simulink model "Simulador_Lazaro_RL_F3"  (R2025b)
settings: Solver=ode45  StartTime=0.0  StopTime=6  FixedStep=0.02  RelTol=1e-3  EnableMultiTasking=on
model workspace (MAT data in the model file):
  ActorLearningRate = 0.0001
  CHECKPOINT_EVERY = 100
  CriticLearningRate = 0.0007
  Expl_Decay = 1.155238628e-05
  Expl_LowerLim = -0.5
  Expl_Mean = 0
  Expl_StdDev = 0.2
  Expl_StdDevMin = 0.05
  Expl_Steps = 120000
  Expl_UpperLim = 0.5
  IdRewardList = {'17'}
  LearningRate = 0.0001
  MAX_STEPS_EPISODE = 600
  SampleTime = 0.01
  ScenarioID = '32'
  TOTAL_EPISODES = 200
  Tgt_Decay = 0
  Tgt_LowerLim = -0.5
  Tgt_Mean = 0
  Tgt_StdDev = 0.2
  Tgt_StdDevMin = 0.2
  Tgt_Steps = 0
  Tgt_UpperLim = 0.5
  Ts = 0.01
  agent : rl.agent.rlTD3Agent (value not decoded)
undefined symbols (not defined in the file): DiscIntNeverNeededParam
libraries: simulink x2, rllib x1, slpidlib x1
settings Model: Creator=Administrador  LastModifiedBy=richards
settings SolverCC: ZeroCrossControl=DisableAll  AlgebraicLoopSolver=TrustRegion
settings DataIOCC: LimitDataPoints=on  SaveFormat=Array  SignalLoggingSaveFormat=ModelDataLogs  DSMLogging=off  ReturnWorkspaceOutputs=off
settings OptimizationCC: ExpressionDepthLimit=2147483647.0  LifeSpan=inf
settings DebuggingCC: UnderspecifiedInitializationDetection=Classic  MergeDetectMultiDrivingBlocksExec=none  SolverPrmCheckMsg=warning  FcnCallInpInsideContextMsg=warning  UnconnectedInputMsg=warning  UnconnectedOutputMsg=warning  UnconnectedLineMsg=warning  StateNameClashWarn=warning  SFUnexpectedBacktrackingDiag=warning  SFNoUnconditionalDefaultTransitionDiag=warning  SFExecutionAtInitializationDiag=none  ArithmeticOperatorsInVariantConditions=warning
settings HardwareCC: ProdBitPerPointer=32.0  ProdBitPerSizeT=32.0  ProdBitPerPtrDiffT=32.0  ProdLargestAtomicFloat=None  ProdIntDivRoundTo=Undefined  ProdEndianess=Unspecified  ProdWordSize=32.0  ProdHWDeviceType=32-bit Generic
settings ModelReferenceCC: EnableRefExpFcnMdlSchedulingChecks=on  PropagateSignalLabelsOutOfModel=off
settings SFSimCC: SimUseLocalCustomCode=off  SimParseCustomCode=off  CustomCodeUndefinedFunction=UseInterfaceOnly
settings RTWCC: RTWUseLocalCustomCode=off  GPUComputeCapability=3.5
settings CodeAppCC: StateflowObjectComments=on
settings GRTTargetCC: TargetLangStandard=C89/C90 (ANSI)  CombineOutputUpdateFcns=off  GRTInterface=on
settings ConfigComp: CovMetricSettings=dw  CovSaveCumulativeToWorkspaceVar=on  CovSaveSingleToWorkspaceVar=on  CovExternalEMLEnable=off

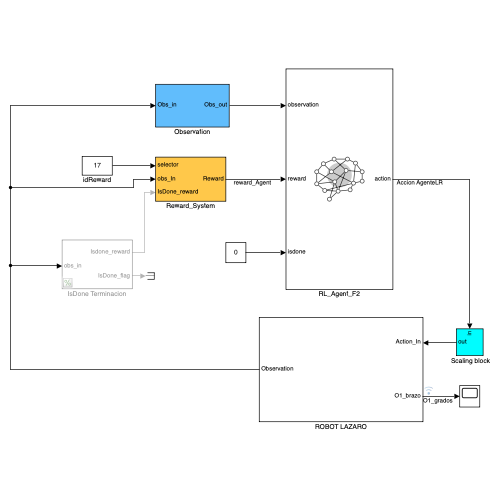
[thumbnail: Simulink's stored preview of the top-level diagram]
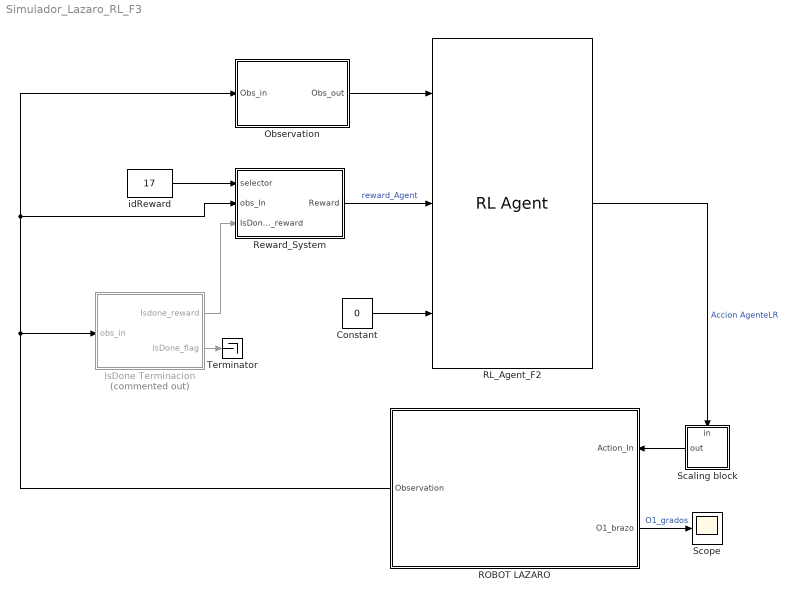
== system Simulador_Lazaro_RL_F3 ==
  " Scaling block" : Subsystem
      settings: PortSchema="{\"entries\":[{\"content\":{\"sides\":[{\"content\":{\"connectorIds\":[\"Out1\"]},\"type\":\"ConnectorPlacement.EquallySpacedRectSide\",\"uuid\":\"a790eab9-2b43-47a4-b062-d830449fd16c\"},{\"content\":{\"side\":\"RIGHT\"},\"type\":\"ConnectorPlacement.EquallySpacedRectSide\",\"uuid\":\"29b5b92f-6241-42c9-b69f-7e34ffae78d0\"},{\"content\":{\"connectorIds\":[\"In1\"],\"side\":\"TOP\"},\"type\":\"ConnectorPlacement.EquallySpacedRectSide\",\"uuid\":\"0d9138d4-6546-416f-9897-aa48eddc1957\"},{\"content\":{\"side\":\"BOTTOM\"},\"type\":\"ConnectorPlacement.EquallySpacedRectSide\",\"uuid\":\"c130b2a4-31b4-4ea6-a38d-9072e793f24d\"}]},\"type\":\"ConnectorPlacement.EquallySpacedConnector\",\"uuid\":\"38341b86-6b15-4731-82e8-a6b530589507\"}],\"packageUris\":[\"http://schema.mathworks.com/mf0/Connector/R2020b\"],\"version\":\"1.0\"}"
  Constant : Constant  Value=0
  IsDone Terminacion : Subsystem  Commented=on
  Observation : Subsystem
      settings: MinAlgLoopOccurrences=on
  RL_Agent_F2 : rllib/RL Agent  Agent=agent  ExternalActionAsInput=off  ProvideLastAction=off  ProvideCumRwd=off  TreatAsDirectFeedthrough=on  UseStrictObservationDataTypes=off
  ROBOT LAZARO : Subsystem
      settings: PortSchema="{\"entries\":[{\"content\":{\"sides\":[{\"content\":{\"connectorIds\":[\"Out2\"]},\"type\":\"ConnectorPlacement.EquallySpacedRectSide\",\"uuid\":\"37102e5d-7d53-4887-99df-ea23d4bb6b5f\"},{\"content\":{\"connectorIds\":[\"In1\",\"Out1\"],\"side\":\"RIGHT\"},\"type\":\"ConnectorPlacement.EquallySpacedRectSide\",\"uuid\":\"afcf0875-52fe-459c-9e77-306d435328c8\"},{\"content\":{\"side\":\"TOP\"},\"type\":\"ConnectorPlacement.EquallySpacedRectSide\",\"uuid\":\"2ef0c81c-cb20-4dc7-992a-873989b99f1b\"}]},\"type\":\"ConnectorPlacement.EquallySpacedConnector\",\"uuid\":\"5a61918d-759d-4f32-b21d-c30e6bf5e1c6\"}],\"packageUris\":[\"http://schema.mathworks.com/mf0/Connector/R2020b\"],\"version\":\"1.0\"}"
  Reward_System : Subsystem
  Scope : Scope
      settings: ActiveDisplayYMinimum=-0.33259239487088316  ActiveDisplayYMaximum=1.9531277507122846  DataLoggingVariableName=ScopeData10
  Terminator : Terminator
  idReward : Constant  Value=17  OutDataTypeStr=uint8
  wires:
  ROBOT LAZARO/2(Observation) -> Observation/1(Obs_in), Reward_System/2(obs_In), IsDone Terminacion/1(obs_in)
  ROBOT LAZARO/1(O1_brazo) -> Scope/1  "O1_grados"
  RL_Agent_F2/1 -> " Scaling block"/1(in)  "Accion AgenteLR"
  " Scaling block"/1(out) -> ROBOT LAZARO/1(Action_In)
  idReward/1 -> Reward_System/1(selector)
  Constant/1 -> RL_Agent_F2/3
  Reward_System/1(Reward) -> RL_Agent_F2/2  "reward_Agent"
  Observation/1(Obs_out) -> RL_Agent_F2/1
  IsDone Terminacion/1(Isdone_reward) -> Reward_System/3(IsDone_reward)
  IsDone Terminacion/2(IsDone_flag) -> Terminator/1

== system Simulador_Lazaro_RL_F3/ Scaling block ==
  note: Saturation:
      | upper: 20
      | lower: -20
  in : Inport
  Constant1 : Constant  Value=0
  K_RL : Gain  Gain=10
  Saturation : Saturate  UpperLimit=20  LowerLimit=-20
  Sum1 : Sum  Inputs=|++
  out : Outport
  wires:
  Sum1/1 -> out/1  "Tt"
  Saturation/1 -> Sum1/1  "K_RL_sat"
  in/1 -> K_RL/1
  Constant1/1 -> Sum1/2
  K_RL/1 -> Saturation/1  "K_RL"

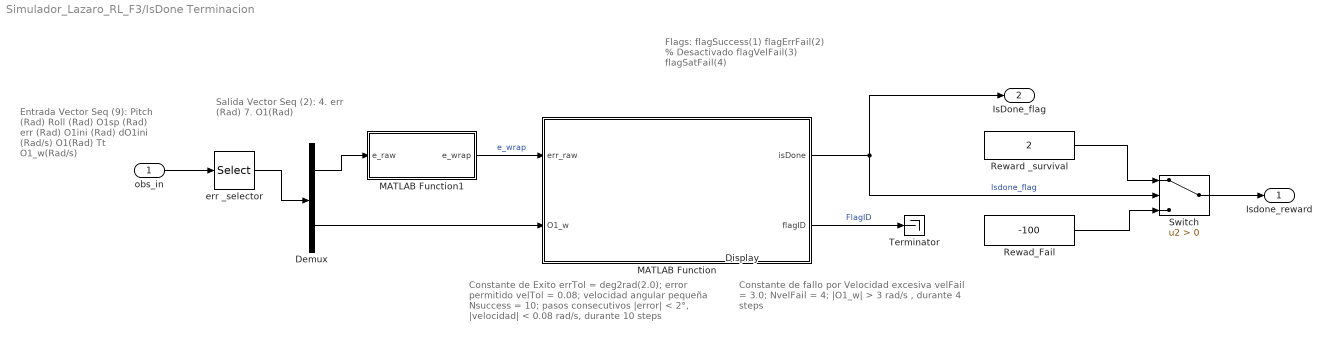
== system Simulador_Lazaro_RL_F3/IsDone Terminacion ==
  note: Flags:
      | flagSuccess(1)
      | flagErrFail(2) % Desactivado
      | flagVelFail(3)
      | flagSatFail(4)
  note: Constante de Exito
      |
      | errTol = deg2rad(2.0); error permitido
      | velTol = 0.08; velocidad angular pequeña
      | Nsuccess = 10; pasos consecutivos
      |
      | |error| < 2°, |velocidad| < 0.08 rad/s, durante 10 steps
  note: Constante de fallo por Velocidad excesiva
      |
      | velFail = 3.0;
      | NvelFail = 4;
      |
      | |O1_w| > 3 rad/s , durante 4 steps
  note: Entrada
      | Vector Seq (9):
      | Pitch (Rad)
      | Roll (Rad)
      | O1sp (Rad)
      | err (Rad)
      | O1ini (Rad)
      | dO1ini (Rad/s)
      | O1(Rad)
      | Tt
      | O1_w(Rad/s)
  note: Salida
      | Vector Seq (2):
      | 4. err (Rad)
      | 7. O1(Rad)
  obs_in : Inport
  Demux : Demux  Outputs=2
  Display : DisplayBlock  LabelPosition=Hide  Transparency=1  GridColor=[0.502, 0.502, 0.502]
  MATLAB Function : MATLAB Function  TreatAsAtomicUnit=on
  MATLAB Function1 : MATLAB Function  TreatAsAtomicUnit=on
  Rewad_Fail : Constant  Value=-100
  Reward _survival : Constant  Value=2
  Switch : Switch  Criteria=u2 > Threshold  SaturateOnIntegerOverflow=off  InputSameDT=off
  Terminator : Terminator
  err _selector : Selector  InputPortWidth=9  IndexOptions=Index vector (dialog)  Indices=[4 7]  OutputSizes=1
  Isdone_reward : Outport
  IsDone_flag : Outport  Port=2
  wires:
  obs_in/1 -> err _selector/1
  err _selector/1 -> Demux/1
  MATLAB Function1/1(e_wrap) -> MATLAB Function/1(err_raw)  "e_wrap"
  Rewad_Fail/1 -> Switch/3
  Switch/1 -> Isdone_reward/1
  MATLAB Function/1(isDone) -> IsDone_flag/1, Switch/2  "Isdone_flag"
  Reward _survival/1 -> Switch/1
  Demux/1 -> MATLAB Function1/1(e_raw)
  Demux/2 -> MATLAB Function/2(O1_w)
  MATLAB Function/2(flagID) -> Terminator/1  "FlagID"

== system Simulador_Lazaro_RL_F3/Observation ==
  note: Entrada:
  note: Vector de Observation:
      | Vector Seq (14):
      |
      | Pitch (sin)
      | Pitch (cos)
      | Roll (sin)
      | Roll (cos)
      | O1sp (sin)
      | O1sp (cos)
      | err (sin) / wrapeado
      | err (cos) / wrapeado
      | O1ini (sin)
      | O1ini (cos)
      | dO1ini (rad/s)
      | O1(sin)
      | O1(cos)
      | O1_w(Rad/s)
  note: Salida:
  note: Vector Seq (9):
      | Pitch (Rad)
      | Roll (Rad)
      | O1sp (Rad)
      | err (Rad)
      | O1ini (Rad)
      | dO1ini (Rad/s)
      | O1(Rad)
      | Tt
      | O1_vel(Rad/s)
  note: Vector Seq (5):
      | 1. Pitch (Rad)
      | 2. Roll (Rad)
      | 3. O1sp (Rad)
      | 5. O1ini (Rad)
      | 7. O1(Rad)
  note: Vector de Observation:
      | Vector Seq (2):
      | 6. dO1ini (Rad/s)
      | 9. O1_w(Rad/s)
  note: Salida:
      | Vector Seq (1):
      | 4. err (Rad)
  Obs_in : Inport
  Demux : Demux  Outputs=2
  MATLAB Function2 : MATLAB Function  TreatAsAtomicUnit=on
  Memory : Memory
  Selector : Selector  InputPortWidth=9  IndexOptions=Index vector (dialog)  Indices=[1 2 3 5 7]  OutputSizes=1
  Selector1 : Selector  InputPortWidth=9  IndexOptions=Index vector (dialog)  Indices=[6 9]  OutputSizes=1
  Selector2 : Selector  InputPortWidth=9  IndexOptions=Index vector (dialog)  Indices=[4]  OutputSizes=1
  Obs_out : Outport
  wires:
  Selector/1 -> MATLAB Function2/1(u_select)
  Obs_in/1 -> Selector/1, Selector1/1, Selector2/1
  Selector2/1 -> MATLAB Function2/2(err_raw)
  Selector1/1 -> Demux/1
  Demux/2 -> MATLAB Function2/4(O1_w)
  Demux/1 -> MATLAB Function2/3(dO1ini)
  MATLAB Function2/1(obs) -> Memory/1
  Memory/1 -> Obs_out/1

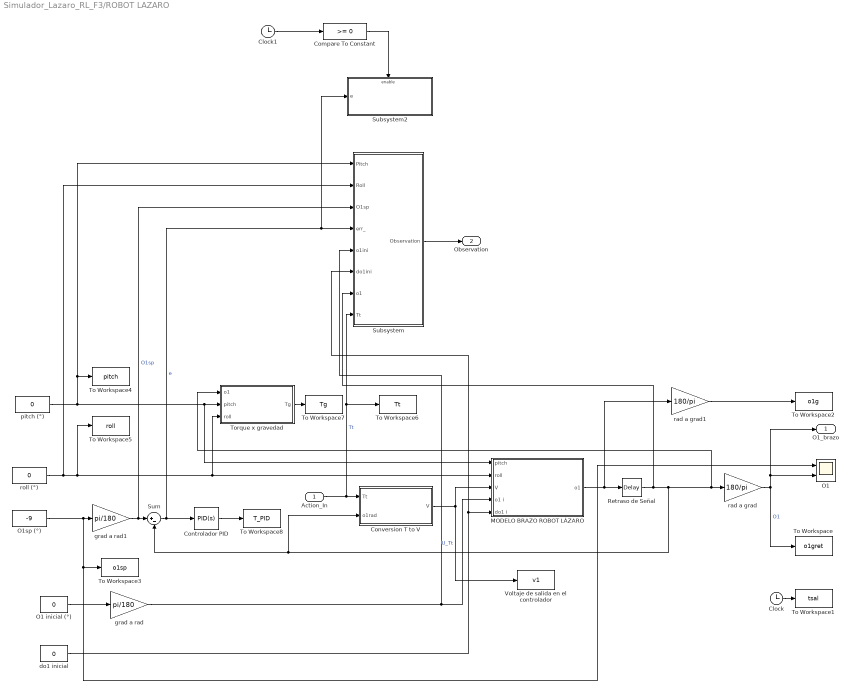
== system Simulador_Lazaro_RL_F3/ROBOT LAZARO ==
  Action_In : Inport
  Clock : Clock
  Clock1 : Clock
  Compare To Constant : simulink/Logic and Bit Operations/Compare To Constant  relop=>=  const=0  OutDataTypeStr=boolean
  Controlador PID : slpidlib/PID Controller  Controller=PID  Form=Parallel  TimeDomain=Continuous-time  UseExternalTs=off  SampleTime=1  IntegratorMethod=Forward Euler  FilterMethod=Forward Euler  ControllerParametersSource=internal  P=5  I=0.2  UseKiTs=off  D=2  UseExternalDerivativeSource=off  N=100  UseFilter=on  TunerSelectOption=Transfer Function Based (PID Tuner App)  InitialConditionSource=internal  InitialConditionForIntegrator=0  InitialConditionForFilter=0  DifferentiatorICPrevScaledInput=0  ExternalReset=none  TrackingMode=off  Kt=1  LimitOutput=off  SatLimitsSource=internal  UpperSaturationLimit=inf  LowerSaturationLimit=-inf  LinearizeAsGain=off  AntiWindupMode=none  Kb=1  LimitIntegrator=off  UpperIntegratorSaturationLimit=inf  LowerIntegratorSaturationLimit=-inf  POutMin=[]  POutMax=[]  IOutMin=[]  IOutMax=[]  DOutMin=[]  DOutMax=[]  NOutMin=[]  NOutMax=[]  SumOutMin=[]  SumOutMax=[]  SaturationOutMin=[]  SaturationOutMax=[]  PParamMin=[]  PParamMax=[]  IParamMin=[]  IParamMax=[]  DParamMin=[]  DParamMax=[]  NParamMin=[]  NParamMax=[]  KbParamMin=[]  KbParamMax=[]  KtParamMin=[]  KtParamMax=[]  KbOutMin=[]  KbOutMax=[]  KtOutMin=[]  KtOutMax=[]  IntegratorOutMin=[]  IntegratorOutMax=[]  FilterOutMin=[]  FilterOutMax=[]  SumI1OutMin=[]  SumI1OutMax=[]  SumI2OutMin=[]  SumI2OutMax=[]  SumI3OutMin=[]  SumI3OutMax=[]  SumI4OutMin=[]  SumI4OutMax=[]  SumDOutMin=[]  SumDOutMax=[]  DifferentiatorOutMin=[]  DifferentiatorOutMax=[]  FilterDiffOutCoefMin=[]  FilterDiffOutCoefMax=[]  ReciprocalOutMin=[]  ReciprocalOutMax=[]  SumDenOutMin=[]  SumDenOutMax=[]  SumNumOutMin=[]  SumNumOutMax=[]  DivideOutMin=[]  DivideOutMax=[]  UdiffTsProdOutMin=[]  UdiffTsProdOutMax=[]  NTsProdOutMin=[]  NTsProdOutMax=[]  UintegralTsProdOutMin=[]  UintegralTsProdOutMax=[]  UngainTsProdOutMin=[]  UngainTsProdOutMax=[]  ClampingZeroOutDataTypeStr=Inherit: Inherit via back propagation  ClampingZeroOutMin=[]  ClampingZeroOutMax=[]  FilterDenConstantOutDataTypeStr=Inherit: Inherit via back propagation  FilterDenConstantOutMin=[]  FilterDenConstantOutMax=[]  PCopyOutDataTypeStr=Inherit: Inherit via back propagation  PCopyOutMin=[]  PCopyOutMax=[]  NCopyOutDataTypeStr=Inherit: Inherit via back propagation  NCopyOutMin=[]  NCopyOutMax=[]  IntegratorContinuousStateAttributes=''  IntegratorStateMustResolveToSignalObject=off  IntegratorRTWStateStorageClass=Auto  FilterContinuousStateAttributes=''  FilterStateMustResolveToSignalObject=off  FilterRTWStateStorageClass=Auto  ParallelPVariant=InternalParameters  IdealPVariant=Disabled  IVariant=InternalParameters  DVariant=InternalParameters  IntegratorVariant=Continuous  SatVariant=Disabled  AWVariant=Passthrough  PCopyVariant=Disabled  TRVariant=Disabled  FdbkBlocksVariant=Forward  IdealPFdbkVariant=Disabled  SatFdbkVariant=Disabled  DerivativeFilterVariant=ContFilter  NVariant=InternalParameters  NCopyVariant=Disabled  FilterICVariant=InternalICsFilter  IntegratorICVariant=InternalICs  ExternalResetVariant=Disabled  TRSumVariant=Passthrough  SumFdbkVariant=Disabled  SumVariant=Sum_PID  TsampFilterVariant=InternalTs  TsampNgainVariant=Passthrough  TsampIntegralVariant=Disabled_SignalSpec  ExternalSaturationOutDataTypeStr=Inherit: Same as second input  DerivativeSourceVariant=ErrorSignal  PreIntVariant=InternalPreInt
  Conversion T to V : Subsystem
  MODELO BRAZO ROBOT LÁZARO : Subsystem
  O1 : Scope  NumInputPorts=2
      settings: ActiveDisplayYMinimum=-3.4920229278516364  ActiveDisplayYMaximum=30.38800254753907  DataLoggingMaxPoints=50000
  O1 inicial (°) : Constant  Value=0
  O1sp (°) : Constant  Value=-9  OutDataTypeStr=Inherit: Inherit via back propagation
  Retraso de Señal : TransportDelay  DelayTime=0
  Subsystem : Subsystem
  Subsystem2 : Subsystem
  Sum : Sum  Inputs=|+-
  To Workspace : ToWorkspace  VariableName=o1gret  SampleTime=-1
  To Workspace1 : ToWorkspace  VariableName=tsal  SampleTime=-1
  To Workspace2 : ToWorkspace  VariableName=o1g  SampleTime=-1
  To Workspace3 : ToWorkspace  VariableName=o1sp  SampleTime=-1
  To Workspace4 : ToWorkspace  VariableName=pitch  SampleTime=-1
  To Workspace5 : ToWorkspace  VariableName=roll  SampleTime=-1
  To Workspace6 : ToWorkspace  VariableName=Tt  SaveFormat=Timeseries  SampleTime=-1
  To Workspace7 : ToWorkspace  VariableName=Tg  SaveFormat=Timeseries  SampleTime=-1
  To Workspace8 : ToWorkspace  VariableName=T_PID  SaveFormat=Timeseries  SampleTime=-1
  Torque x gravedad : Subsystem
  Voltaje de salida en el controlador : ToWorkspace  VariableName=v1  SampleTime=-1
  "do1 inicial " : Constant  Value=0
  grad a rad : Gain  Gain=pi/180
  grad a rad1 : Gain  Gain=pi/180
  pitch (°) : Constant  Value=0
  rad a grad : Gain  Gain=180/pi
  rad a grad1 : Gain  Gain=180/pi
  roll (°) : Constant  Value=0
  O1_brazo : Outport
  Observation : Outport  Port=2
  wires:
  "do1 inicial "/1 -> Subsystem/6(do1ini), MODELO BRAZO ROBOT LÁZARO/5(do1 i)
  Controlador PID/1 -> To Workspace8/1
  Clock/1 -> To Workspace1/1
  Torque x gravedad/1(Tg) -> To Workspace7/1
  Action_In/1 -> Subsystem/8(Tt), To Workspace6/1, Conversion T to V/1(Tt)  "Tt"
  grad a rad1/1 -> Subsystem/3(O1sp), Sum/1  "O1sp"
  O1 inicial (°)/1 -> grad a rad/1
  MODELO BRAZO ROBOT LÁZARO/1(o1) -> Retraso de Señal/1, rad a grad1/1
  grad a rad/1 -> Subsystem/5(o1ini), MODELO BRAZO ROBOT LÁZARO/4(o1 i)
  rad a grad/1 -> To Workspace/1, O1/2, O1_brazo/1  "O1"
  pitch (°)/1 -> Subsystem/1(Pitch), To Workspace4/1, Torque x gravedad/2(pitch), MODELO BRAZO ROBOT LÁZARO/1(pitch)
  Retraso de Señal/1 -> Subsystem/7(o1), rad a grad/1, Torque x gravedad/1(o1), Sum/2, Conversion T to V/2(o1rad)
  Conversion T to V/1(V) -> MODELO BRAZO ROBOT LÁZARO/3(V), Voltaje de salida en el controlador/1  "U_Tt"
  O1sp (°)/1 -> grad a rad1/1, To Workspace3/1, O1/1
  roll (°)/1 -> Subsystem/2(Roll), To Workspace5/1, Torque x gravedad/3(roll), MODELO BRAZO ROBOT LÁZARO/2(roll)
  rad a grad1/1 -> To Workspace2/1
  Sum/1 -> Controlador PID/1, Subsystem2/1(e), Subsystem/4(err_)  "e"
  Subsystem/1(Observation) -> Observation/1
  Clock1/1 -> Compare To Constant/1
  Compare To Constant/1 -> Subsystem2/Enable

== system Simulador_Lazaro_RL_F3/Reward_System ==
  note: Signal _out (4) : err (Rad)
  note: Signal _out (8) : Tt
  note: Signal _out (9) : O1_w
  note: Vector Seq (9):
      | Pitch (Rad)
      | Roll (Rad)
      | O1sp (Rad)
      | err (Rad)
      | O1ini (Rad)
      | dO1ini (Rad/s)
      | O1(Rad)
      | Tt
      | O1_w(Rad/s)
  selector : Inport
  obs_In : Inport  Port=2
  IsDone_reward : Inport  Port=3
  Compare To Constant17 : simulink/Logic and Bit Operations/Compare To Constant  relop===  const=17  OutDataTypeStr=boolean
  Subsystem18 : Subsystem
  Sum : Sum  Inputs=|++
  Tt_selector : Selector  InputPortWidth=9  IndexOptions=Index vector (dialog)  Indices=8  OutputSizes=1
  Tt_selector1 : Selector  InputPortWidth=9  IndexOptions=Index vector (dialog)  Indices=9  OutputSizes=1
  err _selector : Selector  InputPortWidth=9  IndexOptions=Index vector (dialog)  Indices=4  OutputSizes=1
  Reward : Outport
  wires:
  selector/1 -> Compare To Constant17/1
  obs_In/1 -> err _selector/1, Tt_selector1/1, Tt_selector/1
  Tt_selector/1 -> Subsystem18/2(Signal T)  "Tt_vector"
  Subsystem18/1(Reward signal) -> Sum/1
  Compare To Constant17/1 -> Subsystem18/Enable
  Tt_selector1/1 -> Subsystem18/3(O1_w)  "O1_w"
  err _selector/1 -> Subsystem18/1(error)  "Err_vector"
  Sum/1 -> Reward/1
  IsDone_reward/1 -> Sum/2

== system Simulador_Lazaro_RL_F3/ROBOT LAZARO/Conversion T to V ==
  Tt : Inport
  o1rad : Inport  Port=2
  1/Kp : Gain  Gain=1/1.25
  Derivative : Derivative
  Kb : Gain  Gain=0.79
  R : Gain  Gain=3.1
  Saturation : Saturate  UpperLimit=6  LowerLimit=-6
  Sum : Sum  Inputs=|++
  mg1 : Gain  Gain=3
  mg2 : Gain  Gain=1/3
  V : Outport
  wires:
  o1rad/1 -> mg1/1
  mg1/1 -> Derivative/1
  Derivative/1 -> Kb/1
  Tt/1 -> mg2/1
  R/1 -> 1/Kp/1
  1/Kp/1 -> Sum/1
  Kb/1 -> Sum/2
  Sum/1 -> Saturation/1
  mg2/1 -> R/1
  Saturation/1 -> V/1

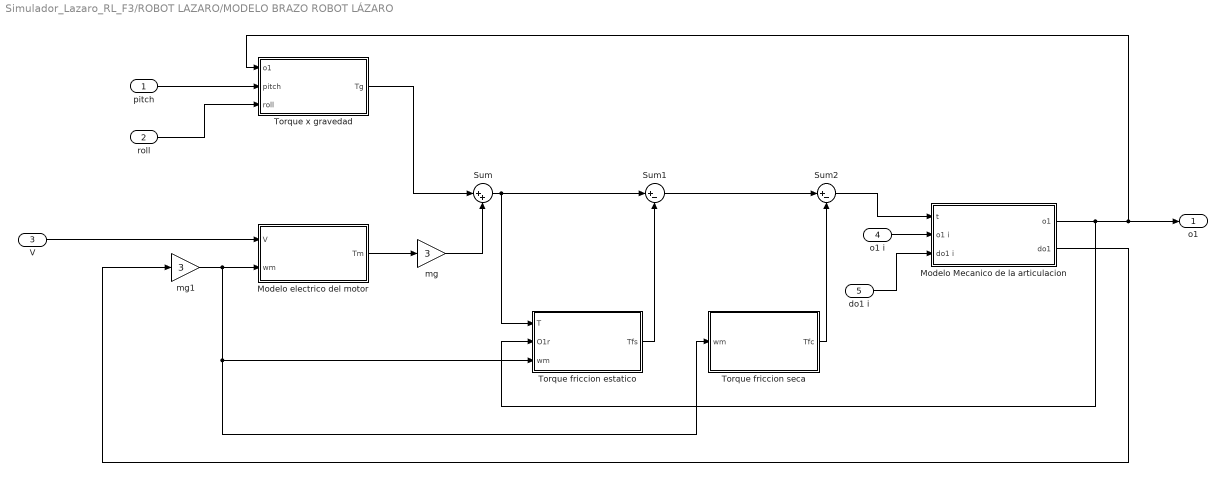
== system Simulador_Lazaro_RL_F3/ROBOT LAZARO/MODELO BRAZO ROBOT LÁZARO ==
  pitch : Inport
  roll : Inport  Port=2
  V : Inport  Port=3
  o1 i : Inport  Port=4
  do1 i : Inport  Port=5
  Modelo Mecanico de la articulacion : Subsystem
  Modelo electrico del motor : Subsystem
  Sum : Sum  Inputs=|++
  Sum1 : Sum  Inputs=|+-
  Sum2 : Sum  Inputs=|+-
  Torque friccion estatico : Subsystem
  Torque friccion seca : Subsystem
  Torque x gravedad : Subsystem
  mg : Gain  Gain=3
  mg1 : Gain  Gain=3
  o1 : Outport
  wires:
  pitch/1 -> Torque x gravedad/2(pitch)
  roll/1 -> Torque x gravedad/3(roll)
  Modelo Mecanico de la articulacion/1(o1) -> o1/1, Torque x gravedad/1(o1), Torque friccion estatico/2(O1r)
  Sum/1 -> Sum1/1, Torque friccion estatico/1(T)
  Torque x gravedad/1(Tg) -> Sum/1
  Modelo electrico del motor/1(Tm) -> mg/1
  Modelo Mecanico de la articulacion/2(do1) -> mg1/1
  V/1 -> Modelo electrico del motor/1(V)
  mg1/1 -> Modelo electrico del motor/2(wm), Torque friccion estatico/3(wm), Torque friccion seca/1(wm)
  o1 i/1 -> Modelo Mecanico de la articulacion/2(o1 i)
  do1 i/1 -> Modelo Mecanico de la articulacion/3(do1 i)
  Sum1/1 -> Sum2/1
  Sum2/1 -> Modelo Mecanico de la articulacion/1(t)
  Torque friccion estatico/1(Tfs) -> Sum1/2
  Torque friccion seca/1(Tfc) -> Sum2/2
  mg/1 -> Sum/2

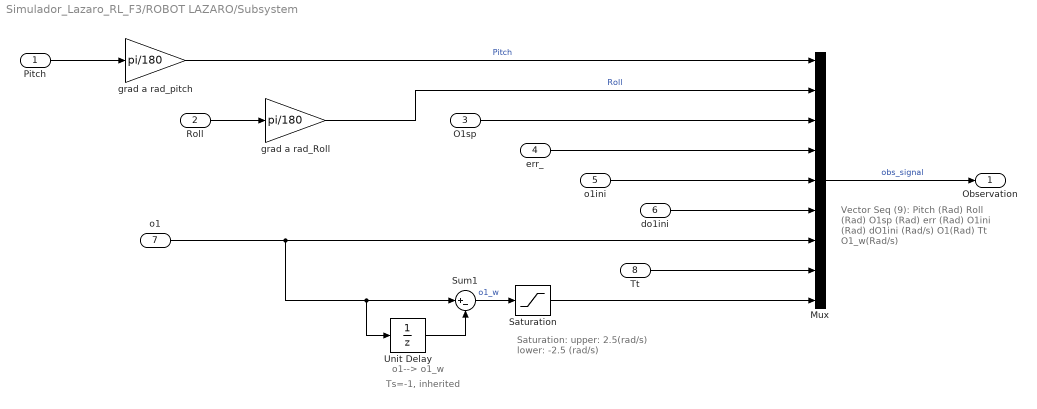
== system Simulador_Lazaro_RL_F3/ROBOT LAZARO/Subsystem ==
  note: Saturation:
      | upper: 2.5(rad/s)
      | lower: -2.5 (rad/s)
  note: o1--> o1_w
  note: Vector Seq (9):
      | Pitch (Rad)
      | Roll (Rad)
      | O1sp (Rad)
      | err (Rad)
      | O1ini (Rad)
      | dO1ini (Rad/s)
      | O1(Rad)
      | Tt
      | O1_w(Rad/s)
  note: Ts=-1, inherited
  Pitch : Inport
  Roll : Inport  Port=2
  O1sp : Inport  Port=3
  err_ : Inport  Port=4
  o1ini : Inport  Port=5
  do1ini : Inport  Port=6
  o1 : Inport  Port=7
  Tt : Inport  Port=8
  Mux : Mux  Inputs=9
  Saturation : Saturate  UpperLimit=2.5  LowerLimit=-2.5
  Sum1 : Sum  Inputs=|+-
  Unit Delay : UnitDelay  SampleTime=-1  HasFrameUpgradeWarning=on
  grad a rad_Roll : Gain  Gain=pi/180
  grad a rad_pitch : Gain  Gain=pi/180
  Observation : Outport
  wires:
  Pitch/1 -> grad a rad_pitch/1
  Roll/1 -> grad a rad_Roll/1
  o1ini/1 -> Mux/5
  err_/1 -> Mux/4
  grad a rad_Roll/1 -> Mux/2  "Roll"
  Sum1/1 -> Saturation/1  "o1_w"
  O1sp/1 -> Mux/3
  Mux/1 -> Observation/1  "obs_signal"
  o1/1 -> Sum1/1, Unit Delay/1, Mux/7
  grad a rad_pitch/1 -> Mux/1  "Pitch"
  Saturation/1 -> Mux/9
  do1ini/1 -> Mux/6
  Tt/1 -> Mux/8
  Unit Delay/1 -> Sum1/2

== system Simulador_Lazaro_RL_F3/ROBOT LAZARO/Subsystem2 ==
  e : Inport
  Enable : EnablePort
  Data Store Write : DataStoreWrite  IndexOptions=Index vector (dialog)  Indices=1  OutputSizes=1
  Gain : Gain  Gain=180/pi
  MATLAB Function1 : MATLAB Function  TreatAsAtomicUnit=on
  RMSE_last : DataStoreMemory  WriteAfterWriteMsg=warning  ShowAdditionalParam=off
  Subsystem1 : Subsystem
  Subsystem2 : Subsystem
  Terminator : Terminator
  To Workspace9 : ToWorkspace  VariableName=RMSE_last  SampleTime=-1
      settings: MaxDataPoints=1
  Unit Delay : UnitDelay  SampleTime=-1  HasFrameUpgradeWarning=on
  wires:
  e/1 -> Gain/1
  Unit Delay/1 -> To Workspace9/1, Data Store Write/1
  Subsystem1/1(rmse_episode) -> Unit Delay/1
  MATLAB Function1/1(y) -> Subsystem2/1(e)  "e_warp"
  Subsystem2/1(rmse_episode) -> Terminator/1  "RMSE_wrap (°)"
  Gain/1 -> Subsystem1/1(e), MATLAB Function1/1(err_raw)

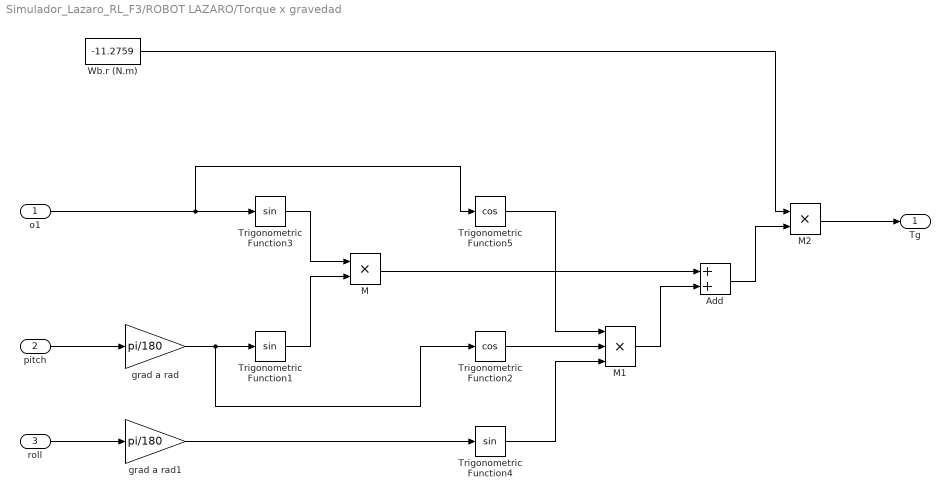
== system Simulador_Lazaro_RL_F3/ROBOT LAZARO/Torque x gravedad ==
  o1 : Inport
  pitch : Inport  Port=2
  roll : Inport  Port=3
  Add : Sum
  M : Product  Inputs=**
  M1 : Product  Inputs=***
  M2 : Product  Inputs=**
  Trigonometric Function1 : Trigonometry
  Trigonometric Function2 : Trigonometry  Operator=cos
  Trigonometric Function3 : Trigonometry
  Trigonometric Function4 : Trigonometry
  Trigonometric Function5 : Trigonometry  Operator=cos
  Wb.r (N.m) : Constant  Value=-11.2759
  grad a rad : Gain  Gain=pi/180
  grad a rad1 : Gain  Gain=pi/180
  Tg : Outport
  wires:
  pitch/1 -> grad a rad/1
  grad a rad/1 -> Trigonometric Function1/1, Trigonometric Function2/1
  o1/1 -> Trigonometric Function3/1, Trigonometric Function5/1
  Trigonometric Function1/1 -> M/2
  Trigonometric Function3/1 -> M/1
  Trigonometric Function2/1 -> M1/2
  Trigonometric Function4/1 -> M1/3
  Trigonometric Function5/1 -> M1/1
  M/1 -> Add/1
  M1/1 -> Add/2
  Add/1 -> M2/2
  Wb.r (N.m)/1 -> M2/1
  M2/1 -> Tg/1
  roll/1 -> grad a rad1/1
  grad a rad1/1 -> Trigonometric Function4/1

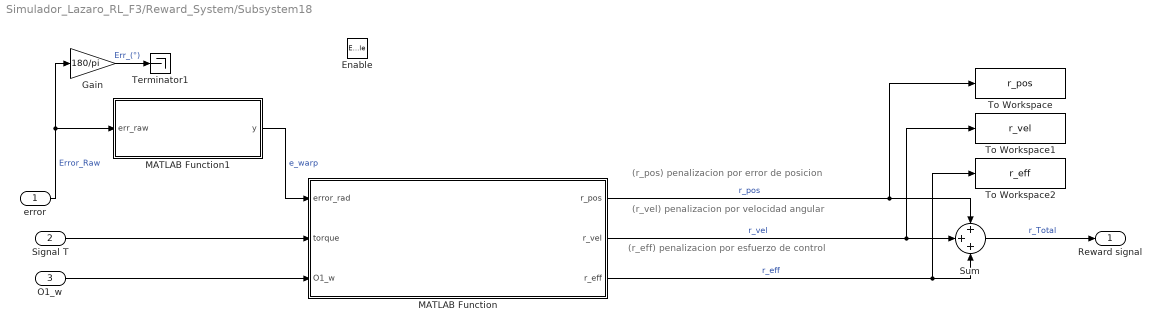
== system Simulador_Lazaro_RL_F3/Reward_System/Subsystem18 ==
  note: (r_eff)
      | penalizacion por esfuerzo de control
  note: (r_vel)
      | penalizacion por velocidad angular
  note: (r_pos)
      | penalizacion por error de posicion
  error : Inport
  Signal T : Inport  Port=2
  O1_w : Inport  Port=3
  Enable : EnablePort
  Gain : Gain  Gain=180/pi
  MATLAB Function : MATLAB Function  TreatAsAtomicUnit=on
  MATLAB Function1 : MATLAB Function  TreatAsAtomicUnit=on
  Sum : Sum  Inputs=+++
  Terminator1 : Terminator
  To Workspace : ToWorkspace  VariableName=r_pos  SaveFormat=Timeseries  SampleTime=-1
  To Workspace1 : ToWorkspace  VariableName=r_vel  SaveFormat=Timeseries  SampleTime=-1
  To Workspace2 : ToWorkspace  VariableName=r_eff  SaveFormat=Timeseries  SampleTime=-1
  Reward signal : Outport
  wires:
  Signal T/1 -> MATLAB Function/2(torque)
  error/1 -> Gain/1, MATLAB Function1/1(err_raw)  "Error_Raw"
  MATLAB Function/1(r_pos) -> To Workspace/1, Sum/1  "r_pos"
  O1_w/1 -> MATLAB Function/3(O1_w)
  MATLAB Function/2(r_vel) -> To Workspace1/1, Sum/2  "r_vel"
  MATLAB Function/3(r_eff) -> To Workspace2/1, Sum/3  "r_eff"
  Sum/1 -> Reward signal/1  "r_Total"
  MATLAB Function1/1(y) -> MATLAB Function/1(error_rad)  "e_warp"
  Gain/1 -> Terminator1/1  "Err_(°)"

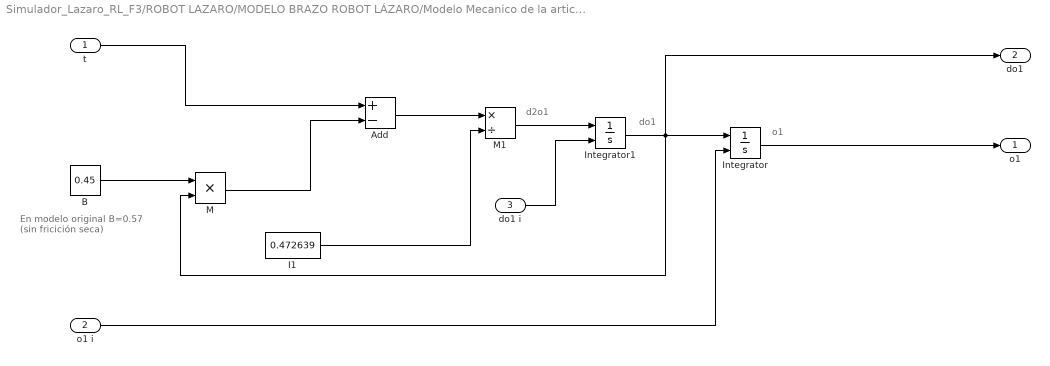
== system Simulador_Lazaro_RL_F3/ROBOT LAZARO/MODELO BRAZO ROBOT LÁZARO/Modelo Mecanico de la articulacion ==
  note: o1
  note: d2o1
  note: do1
  note: En modelo original B=0.57
      | (sin fricición seca)
  t : Inport
  o1 i : Inport  Port=2
  do1 i : Inport  Port=3
  Add : Sum  Inputs=+-
  B : Constant  Value=0.45
  I1 : Constant  Value=0.472639
  Integrator : Integrator  InitialConditionSource=external
  Integrator1 : Integrator  InitialConditionSource=external
  M : Product  Inputs=**
  M1 : Product  Inputs=*/
  o1 : Outport
  do1 : Outport  Port=2
  wires:
  Integrator/1 -> o1/1
  t/1 -> Add/1
  B/1 -> M/1
  M/1 -> Add/2
  Add/1 -> M1/1
  I1/1 -> M1/2
  M1/1 -> Integrator1/1
  Integrator1/1 -> M/2, Integrator/1, do1/1
  o1 i/1 -> Integrator/2
  do1 i/1 -> Integrator1/2

== system Simulador_Lazaro_RL_F3/ROBOT LAZARO/MODELO BRAZO ROBOT LÁZARO/Modelo electrico del motor ==
  V : Inport
  wm : Inport  Port=2
  1/R : Gain  Gain=1/3.1
  Add : Sum  Inputs=+-
  Kb : Gain  Gain=0.79
  Kp : Gain  Gain=1.25
  Tm : Outport
  wires:
  V/1 -> Add/1
  Add/1 -> Kp/1
  Kp/1 -> 1/R/1
  1/R/1 -> Tm/1
  wm/1 -> Kb/1
  Kb/1 -> Add/2

== system Simulador_Lazaro_RL_F3/ROBOT LAZARO/MODELO BRAZO ROBOT LÁZARO/Torque friccion estatico ==
  T : Inport
  O1r : Inport  Port=2
  wm : Inport  Port=3
  Abs2 : Abs  SaturateOnIntegerOverflow=off
  If Action Subsystem2 : Subsystem  TreatAsAtomicUnit=on
  If Action Subsystem3 : Subsystem  TreatAsAtomicUnit=on
  If1 : If  IfExpression=u1<=1e-14
  Merge : Merge
  Tfs : Outport
  wires:
  Abs2/1 -> If1/1
  wm/1 -> Abs2/1, If Action Subsystem2/3(wm)
  T/1 -> If Action Subsystem2/1(T)
  O1r/1 -> If Action Subsystem2/2(O1r)
  If1/1 -> If Action Subsystem2/Action
  If1/2 -> If Action Subsystem3/Action
  If Action Subsystem2/1(Tfs) -> Merge/1
  If Action Subsystem3/1(Tfs) -> Merge/2
  Merge/1 -> Tfs/1

== system Simulador_Lazaro_RL_F3/ROBOT LAZARO/MODELO BRAZO ROBOT LÁZARO/Torque friccion seca ==
  wm : Inport
  Abs2 : Abs  SaturateOnIntegerOverflow=off
  If Action Subsystem2 : Subsystem  TreatAsAtomicUnit=on
  If Action Subsystem3 : Subsystem  TreatAsAtomicUnit=on
  If1 : If  IfExpression=u1>1e-14
  Merge : Merge
  Tfc : Outport
  wires:
  Abs2/1 -> If1/1
  wm/1 -> Abs2/1, If Action Subsystem2/1(wm)
  If1/1 -> If Action Subsystem2/Action
  If1/2 -> If Action Subsystem3/Action
  If Action Subsystem2/1(Tfc) -> Merge/1
  If Action Subsystem3/1(Tfc) -> Merge/2
  Merge/1 -> Tfc/1

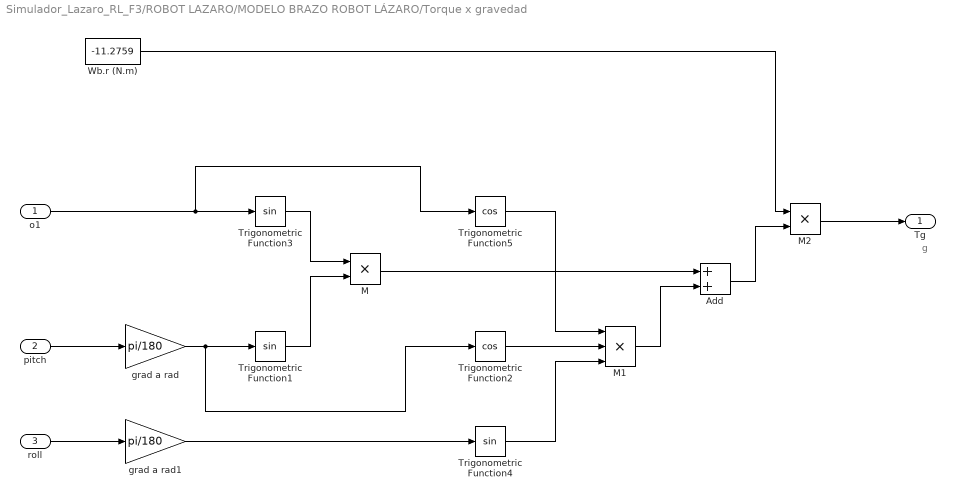
== system Simulador_Lazaro_RL_F3/ROBOT LAZARO/MODELO BRAZO ROBOT LÁZARO/Torque x gravedad ==
  note: g
  o1 : Inport
  pitch : Inport  Port=2
  roll : Inport  Port=3
  Add : Sum
  M : Product  Inputs=**
  M1 : Product  Inputs=***
  M2 : Product  Inputs=**
  Trigonometric Function1 : Trigonometry
  Trigonometric Function2 : Trigonometry  Operator=cos
  Trigonometric Function3 : Trigonometry
  Trigonometric Function4 : Trigonometry
  Trigonometric Function5 : Trigonometry  Operator=cos
  Wb.r (N.m) : Constant  Value=-11.2759
  grad a rad : Gain  Gain=pi/180
  grad a rad1 : Gain  Gain=pi/180
  Tg : Outport
  wires:
  pitch/1 -> grad a rad/1
  grad a rad/1 -> Trigonometric Function1/1, Trigonometric Function2/1
  o1/1 -> Trigonometric Function3/1, Trigonometric Function5/1
  Trigonometric Function1/1 -> M/2
  Trigonometric Function3/1 -> M/1
  Trigonometric Function2/1 -> M1/2
  Trigonometric Function4/1 -> M1/3
  Trigonometric Function5/1 -> M1/1
  M/1 -> Add/1
  M1/1 -> Add/2
  Add/1 -> M2/2
  Wb.r (N.m)/1 -> M2/1
  M2/1 -> Tg/1
  roll/1 -> grad a rad1/1
  grad a rad1/1 -> Trigonometric Function4/1

== system Simulador_Lazaro_RL_F3/ROBOT LAZARO/Subsystem2/Subsystem1 ==
  note: RMSE Calculator (for Train)
  e : Inport
  Constant : Constant
  Discrete-Time Integrator : DiscreteIntegrator  SampleTime=0.01  ICPrevOutput=DiscIntNeverNeededParam  ICPrevScaledInput=DiscIntNeverNeededParam
  Discrete-Time steps counter : DiscreteIntegrator  SampleTime=0.01  ICPrevOutput=DiscIntNeverNeededParam  ICPrevScaledInput=DiscIntNeverNeededParam
  Divide : Product  Inputs=*/
  Math Function : Math  Operator=square
  MinMax : MinMax  Function=max
  Sqrt : Sqrt
  rmse_episode : Outport
  wires:
  e/1 -> Math Function/1
  Sqrt/1 -> rmse_episode/1  "RMSE_clasico (°)"
  Discrete-Time Integrator/1 -> Divide/1  "sum_e2"
  Constant/1 -> Discrete-Time steps counter/1
  Divide/1 -> Sqrt/1  "mean"
  Discrete-Time steps counter/1 -> MinMax/1  "N"
  Math Function/1 -> Discrete-Time Integrator/1
  MinMax/1 -> Divide/2  "n_Safe"

== system Simulador_Lazaro_RL_F3/ROBOT LAZARO/Subsystem2/Subsystem2 ==
  note: RMSE Calculator (for Train)
  e : Inport
  Constant : Constant
  Discrete-Time Integrator : DiscreteIntegrator  SampleTime=0.01  ICPrevOutput=DiscIntNeverNeededParam  ICPrevScaledInput=DiscIntNeverNeededParam
  Discrete-Time steps counter : DiscreteIntegrator  SampleTime=0.01  ICPrevOutput=DiscIntNeverNeededParam  ICPrevScaledInput=DiscIntNeverNeededParam
  Divide : Product  Inputs=*/
  Math Function : Math  Operator=square
  MinMax : MinMax  Function=max
  Sqrt : Sqrt
  rmse_episode : Outport
  wires:
  e/1 -> Math Function/1
  Sqrt/1 -> rmse_episode/1  "rmse_episode"
  Discrete-Time Integrator/1 -> Divide/1  "sum_e2"
  Constant/1 -> Discrete-Time steps counter/1
  Divide/1 -> Sqrt/1  "mean"
  Discrete-Time steps counter/1 -> MinMax/1  "N"
  Math Function/1 -> Discrete-Time Integrator/1
  MinMax/1 -> Divide/2  "n_Safe"

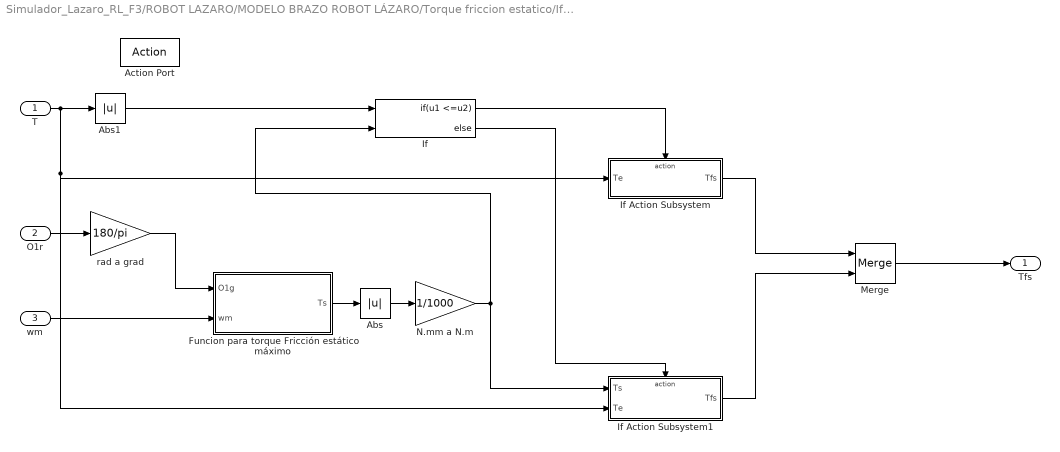
== system Simulador_Lazaro_RL_F3/ROBOT LAZARO/MODELO BRAZO ROBOT LÁZARO/Torque friccion estatico/If Action Subsystem2 ==
  T : Inport
  O1r : Inport  Port=2
  wm : Inport  Port=3
  Action Port : ActionPort  ActionPortLabel=if(u1<=1e-14)
  Abs : Abs  SaturateOnIntegerOverflow=off
  Abs1 : Abs  SaturateOnIntegerOverflow=off
  Funcion para torque Fricción estático máximo : Subsystem
  If : If  NumInputs=2  IfExpression=u1 <=u2
  If Action Subsystem : Subsystem  TreatAsAtomicUnit=on
  If Action Subsystem1 : Subsystem  TreatAsAtomicUnit=on
  Merge : Merge
  N.mm a N.m : Gain  Gain=1/1000
  rad a grad : Gain  Gain=180/pi
  Tfs : Outport
  wires:
  O1r/1 -> rad a grad/1
  rad a grad/1 -> Funcion para torque Fricción estático máximo/1(O1g)
  wm/1 -> Funcion para torque Fricción estático máximo/2(wm)
  Funcion para torque Fricción estático máximo/1(Ts) -> Abs/1
  T/1 -> Abs1/1, If Action Subsystem1/2(Te), If Action Subsystem/1(Te)
  Abs1/1 -> If/1
  Abs/1 -> N.mm a N.m/1
  If/1 -> If Action Subsystem/Action
  If/2 -> If Action Subsystem1/Action
  If Action Subsystem/1(Tfs) -> Merge/1
  If Action Subsystem1/1(Tfs) -> Merge/2
  N.mm a N.m/1 -> If/2, If Action Subsystem1/1(Ts)
  Merge/1 -> Tfs/1

== system Simulador_Lazaro_RL_F3/ROBOT LAZARO/MODELO BRAZO ROBOT LÁZARO/Torque friccion estatico/If Action Subsystem3 ==
  Action Port : ActionPort  ActionPortLabel=else
  Constant : Constant  Value=0
  Tfs : Outport  InitialOutput=0
  wires:
  Constant/1 -> Tfs/1

== system Simulador_Lazaro_RL_F3/ROBOT LAZARO/MODELO BRAZO ROBOT LÁZARO/Torque friccion seca/If Action Subsystem2 ==
  wm : Inport
  Action Port : ActionPort  ActionPortLabel=if(u1>1e-14)
  Constant : Constant  Value=0.3
  Product : Product  RndMeth=Zero
  Sign : Signum
  Tfc : Outport
  wires:
  wm/1 -> Sign/1
  Sign/1 -> Product/1
  Constant/1 -> Product/2
  Product/1 -> Tfc/1

== system Simulador_Lazaro_RL_F3/ROBOT LAZARO/MODELO BRAZO ROBOT LÁZARO/Torque friccion seca/If Action Subsystem3 ==
  Action Port : ActionPort  ActionPortLabel=else
  Constant : Constant  Value=0
  Tfc : Outport  InitialOutput=0
  wires:
  Constant/1 -> Tfc/1

== system Simulador_Lazaro_RL_F3/ROBOT LAZARO/MODELO BRAZO ROBOT LÁZARO/Torque friccion estatico/If Action Subsystem2/Funcion para torque Fricción estático máximo ==
  O1g : Inport
  wm : Inport  Port=2
  If : If  IfExpression=u1 >= 0
  If Action Subsystem : Subsystem  TreatAsAtomicUnit=on
  If Action Subsystem1 : Subsystem  TreatAsAtomicUnit=on
  Merge : Merge
  Sign : Signum
  Ts : Outport
  wires:
  wm/1 -> Sign/1
  Sign/1 -> If/1
  If/2 -> If Action Subsystem1/Action
  If Action Subsystem/1(Test) -> Merge/1
  If Action Subsystem1/1(Test) -> Merge/2
  Merge/1 -> Ts/1
  O1g/1 -> If Action Subsystem/1(O1g), If Action Subsystem1/1(O1g)
  If/1 -> If Action Subsystem/Action

== system Simulador_Lazaro_RL_F3/ROBOT LAZARO/MODELO BRAZO ROBOT LÁZARO/Torque friccion estatico/If Action Subsystem2/If Action Subsystem ==
  Te : Inport
  Action Port : ActionPort  ActionPortLabel=if(u1 <=u2)
  Tfs : Outport
  wires:
  Te/1 -> Tfs/1

== system Simulador_Lazaro_RL_F3/ROBOT LAZARO/MODELO BRAZO ROBOT LÁZARO/Torque friccion estatico/If Action Subsystem2/If Action Subsystem1 ==
  Ts : Inport
  Te : Inport  Port=2
  Action Port : ActionPort  ActionPortLabel=else
  Product : Product  RndMeth=Zero
  Sign : Signum
  Tfs : Outport
  wires:
  Ts/1 -> Product/1
  Product/1 -> Tfs/1
  Te/1 -> Sign/1
  Sign/1 -> Product/2

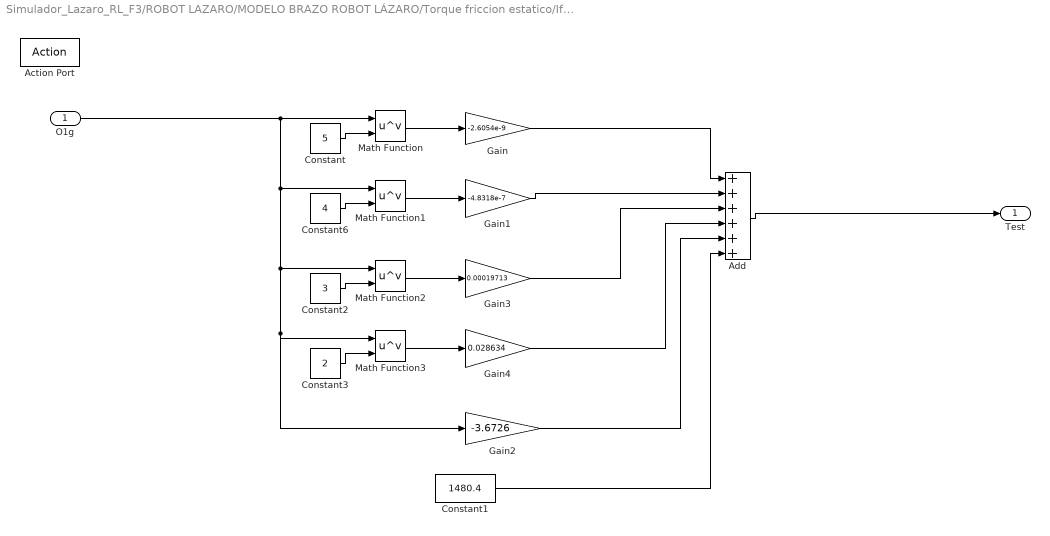
== system Simulador_Lazaro_RL_F3/ROBOT LAZARO/MODELO BRAZO ROBOT LÁZARO/Torque friccion estatico/If Action Subsystem2/Funcion para torque Fricción estático máximo/If Action Subsystem ==
  O1g : Inport
  Action Port : ActionPort  ActionPortLabel=if(u1 >= 0)
  Add : Sum  Inputs=++++++
  Constant : Constant  Value=5
  Constant1 : Constant  Value=1480.4
  Constant2 : Constant  Value=3
  Constant3 : Constant  Value=2
  Constant6 : Constant  Value=4
  Gain : Gain  Gain=-2.6054e-9
  Gain1 : Gain  Gain=-4.8318e-7
  Gain2 : Gain  Gain=-3.6726
  Gain3 : Gain  Gain=0.00019713
  Gain4 : Gain  Gain=0.028634
  Math Function{,1..3} (4 alike) : Math  Operator=pow  SignedPower=on
  Test : Outport
  wires:
  Add/1 -> Test/1
  Constant/1 -> Math Function/2
  Constant6/1 -> Math Function1/2
  Gain/1 -> Add/1
  Gain1/1 -> Add/2
  Math Function/1 -> Gain/1
  Math Function1/1 -> Gain1/1
  O1g/1 -> Math Function/1, Math Function1/1, Math Function2/1, Math Function3/1, Gain2/1
  Constant2/1 -> Math Function2/2
  Math Function2/1 -> Gain3/1
  Constant3/1 -> Math Function3/2
  Math Function3/1 -> Gain4/1
  Gain4/1 -> Add/4
  Gain3/1 -> Add/3
  Gain2/1 -> Add/5
  Constant1/1 -> Add/6

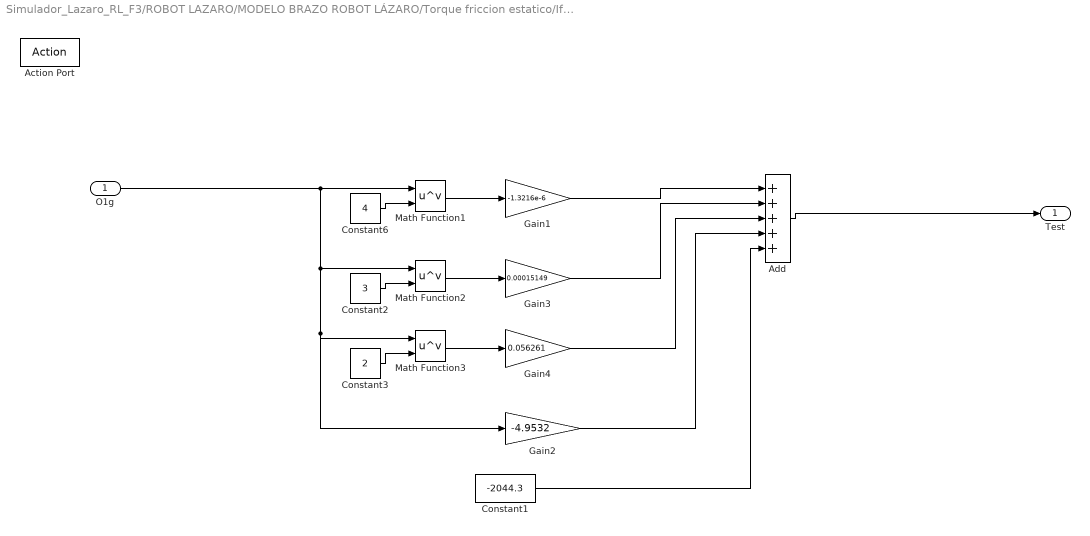
== system Simulador_Lazaro_RL_F3/ROBOT LAZARO/MODELO BRAZO ROBOT LÁZARO/Torque friccion estatico/If Action Subsystem2/Funcion para torque Fricción estático máximo/If Action Subsystem1 ==
  O1g : Inport
  Action Port : ActionPort  ActionPortLabel=else
  Add : Sum  Inputs=+++++
  Constant1 : Constant  Value=-2044.3
  Constant2 : Constant  Value=3
  Constant3 : Constant  Value=2
  Constant6 : Constant  Value=4
  Gain1 : Gain  Gain=-1.3216e-6
  Gain2 : Gain  Gain=-4.9532
  Gain3 : Gain  Gain=0.00015149
  Gain4 : Gain  Gain=0.056261
  Math Function1 : Math  Operator=pow  SignedPower=on
  Math Function2 : Math  Operator=pow  SignedPower=on
  Math Function3 : Math  Operator=pow  SignedPower=on
  Test : Outport
  wires:
  Add/1 -> Test/1
  Constant6/1 -> Math Function1/2
  Math Function1/1 -> Gain1/1
  O1g/1 -> Math Function1/1, Math Function2/1, Math Function3/1, Gain2/1
  Constant2/1 -> Math Function2/2
  Math Function2/1 -> Gain3/1
  Constant3/1 -> Math Function3/2
  Math Function3/1 -> Gain4/1
  Constant1/1 -> Add/5
  Gain1/1 -> Add/1
  Gain3/1 -> Add/2
  Gain4/1 -> Add/3
  Gain2/1 -> Add/4

== matlab function Simulador_Lazaro_RL_F3/Reward_System/Subsystem18/MATLAB Function1 ==
  data: y output; err_raw input
function y = fcn(err_raw)
    y = atan2(sin(err_raw), cos(err_raw));
end

== matlab function Simulador_Lazaro_RL_F3/ROBOT LAZARO/Subsystem2/MATLAB Function1 ==
  data: y output; err_raw input
function y = fcn(err_raw)
%#codegen
    y = atan2(sin(err_raw), cos(err_raw));
end

== matlab function Simulador_Lazaro_RL_F3/Reward_System/Subsystem18/MATLAB Function ==
  data: error_rad input; torque input; r_pos output; O1_w input; r_vel output; r_eff output
function [r_pos, r_vel, r_eff] = fcn(error_rad, torque, O1_w)

% --- asegurar escalares ---
error_rad = error_rad(1);
torque    = torque(1);
O1_w      = O1_w(1);

% ===============================
% PESOS
% ===============================
w_pos = 4.0;
w_vel = 0.07;
w_eff = 0.0015;

% ===============================
% COMPONENTES
% ===============================
% D → métrica angular suave (geodésica)
r_pos = - w_pos * (1 - cos(error_rad));

r_vel = - w_vel * (O1_w^2);
r_eff = - w_eff * (torque^2);

% ===============================
% CAST
% ===============================
r_pos = double(r_pos);
r_vel = double(r_vel);
r_eff = double(r_eff);

end

== matlab function Simulador_Lazaro_RL_F3/IsDone Terminacion/MATLAB Function ==
  data: err_raw input; O1_w input; isDone output; flagID output
function [isDone, flagID] = fcn(err_raw, O1_w)
%#codegen

e_wrap = atan2(sin(err_raw), cos(err_raw));

errTol  = deg2rad(2.0);
velTol  = 0.08;
velFail = 3.0;

flagSuccess = (abs(e_wrap) < errTol) && (abs(O1_w) < velTol);
flagVelFail = (abs(O1_w) > velFail);

isDone = flagSuccess || flagVelFail;

flagID = uint8(0);
if flagSuccess
    flagID = uint8(1);
elseif flagVelFail
    flagID = uint8(3);
end
end

== matlab function Simulador_Lazaro_RL_F3/IsDone Terminacion/MATLAB Function1 ==
  data: e_raw input; e_wrap output
function e_wrap = wrapToPi_err(e_raw)
% e_raw: rad
% e_wrap: rad en [-pi, pi]
e_wrap = atan2(sin(e_raw), cos(e_raw));
end

== matlab function Simulador_Lazaro_RL_F3/Observation/MATLAB Function2 ==
  data: u_select input; obs output; err_raw input; dO1ini input; O1_w input
function obs = fcn(u_select,  err_raw, dO1ini, O1_w)
    % u_select: Vector de 5 [Pitch, Roll, O1sp, O1ini, O1]
    
    % 1. Procesar Senos y Cosenos vectorialmente (Muy rápido)
    s = sin(u_select);
    c = cos(u_select);
    
    % 2. Aplicar wrapToPi al error c
    e_w = atan2(sin(err_raw), cos(err_raw));
    
    % 3. Construir el Vector de Observación (14 señales)
    % Siguiendo el orden exacto del diseño Observable
    obs = [ s(1); c(1); ... % 1-2: Pitch
            s(2); c(2); ... % 3-4: Roll
            s(3); c(3); ... % 5-6: O1sp
            sin(e_w); cos(e_w); ... % 7-8: err wrapeado
            s(4); c(4); ... % 9-10: O1ini
            dO1ini;     ... % 11: dO1ini (directo)
            s(5); c(5); ... % 12-13: O1
            O1_w ];       % 14: O1_w (directo)
    obs = obs(:);
end
